simulink model "TosAeb"  (R2021a)
settings: Solver=FixedStepDiscrete  StartTime=0.0  StopTime=inf  FixedStep=0.01  RelTol=1e-3
undefined symbols (not defined in the file or in its folder): ACCModSts, AccSts1, AEB_ObjectMovingOrientation, AEB_ObjectMovingState, CN_BTCActive, CN_CurrentGearR, CN_CurrentGearR_DHT, CN_PTCActive, CN_VDCActive, CN_VSC_EspOff, CN_VSC_FCW_AEB_Error, CN_VSC_FCW_AEB_NoDispay, CN_VSC_FCW_AEB_PerfmDgdtn, CN_VSC_FCW_AEB_TempUnAvlbl, CN_VSC_VehStandstill, CNaccelAvoidMax, CNaccelAvoidMin, CNbrakePedalPress, CNcomfortStrLeftAccelMax, CNcomfortStrLeftAccelMin, CNcomfortStrRightAccelMax, CNcomfortStrRightAccelMin, CNDrvDoorOpen, CNDrvModeEco, CNDrvModeNorm, CNDrvModeOther, CNDrvModeSport, CNDrvSBRreminder, CNNull, CNobjInvalidID, CNPassDoorOpen, CNPassSBRreminder, CNPEBSAccelNegInf, CNPEBSAccelNull, CNPEBSTimeInf, CNPEBSTimeNull, CNSTOPMAX, CNSTOPNULL, CNsubjWidth, CNSwtOFF, CNSwtON, CNTTCMAX, CNTTCNULL, CNVehConfigAEBCycNone, CNVehConfigAEBNone, CNVehConfigAEBPedNone, CNVehConfigFCWNone, CNVehConfigJANone, CollisionSeverity, FALSE, FCWSnvty, JACycObjCalcInfoDefaultParams, JAPedObjCalcInfoDefaultParams, JAVehObjCalcInfoDefaultParams, latCycObjCalcInfoDefaultParams, latPedObjCalcInfoDefaultParams, longObjCalcInfoDefaultParams, ModSts, movingStatus, ObjectType ... (+1112)
libraries: simulink x3031, AEBSFunctionLib x50, Ta_Library x33, AEBSStateMachineLib x23, Mylibrary x12, simulink_extras x7, ObjSelect_lib x3, dspstat3 x2
(part 46 of 102)

== system TosAeb/FcwAebJa_Step_sys/ALG_AEBSystem/ALG_AEBController/AEB_FuncImpManagementApp/FuncImpArbCalc/BrkReadyFuncImpArbCalc/BrkReadyFuncImpArbCalc/FCTB_PrefillFuncImpArbCalc ==
  FCTB_PrefillModStsArb : Inport
  FCTB_PrefillFuncImpAborCond : Inport  Port=2
  Compare To Constant : simulink/Logic and Bit Operations/Compare To Constant  relop=~=  const=ModSts.ActiveState  OutDataTypeStr=boolean
  Compare To Constant1 : simulink/Logic and Bit Operations/Compare To Constant  relop===  const=FALSE  OutDataTypeStr=boolean
  Constant : Constant  Value=ModSts.StandbyState
  Switch : Switch  Criteria=u2 ~= 0
  Switch1 : Switch  Criteria=u2 ~= 0
  FCTB_PrefillFuncImpArb : Outport
  wires:
  FCTB_PrefillModStsArb/1 -> Switch1/1, Switch/1, Compare To Constant/1
  Compare To Constant/1 -> Switch/2
  FCTB_PrefillFuncImpAborCond/1 -> Compare To Constant1/1
  Compare To Constant1/1 -> Switch1/2
  Constant/1 -> Switch1/3
  Switch1/1 -> Switch/3
  Switch/1 -> FCTB_PrefillFuncImpArb/1

== system TosAeb/FcwAebJa_Step_sys/ALG_AEBSystem/ALG_AEBController/AEB_FuncImpManagementApp/FuncImpArbCalc/BrkReadyFuncImpArbCalc/BrkReadyFuncImpArbCalc/JA_PTW_PrefillFuncImpArbCalc ==
  JA_PTW_PrefillModStsArb : Inport
  JA_PTW_PrefillFuncImpAborCond : Inport  Port=2
  Compare To Constant : simulink/Logic and Bit Operations/Compare To Constant  relop=~=  const=ModSts.ActiveState  OutDataTypeStr=boolean
  Compare To Constant1 : simulink/Logic and Bit Operations/Compare To Constant  relop===  const=FALSE  OutDataTypeStr=boolean
  Constant : Constant  Value=ModSts.StandbyState
  Switch : Switch  Criteria=u2 ~= 0
  Switch1 : Switch  Criteria=u2 ~= 0
  JA_PTW_PrefillFuncImpArb : Outport
  wires:
  Switch/1 -> JA_PTW_PrefillFuncImpArb/1
  Switch1/1 -> Switch/3
  Constant/1 -> Switch1/3
  Compare To Constant1/1 -> Switch1/2
  JA_PTW_PrefillFuncImpAborCond/1 -> Compare To Constant1/1
  Compare To Constant/1 -> Switch/2
  JA_PTW_PrefillModStsArb/1 -> Compare To Constant/1, Switch/1, Switch1/1

== system TosAeb/FcwAebJa_Step_sys/ALG_AEBSystem/ALG_AEBController/AEB_FuncImpManagementApp/FuncImpArbCalc/BrkReadyFuncImpArbCalc/BrkReadyFuncImpArbCalc/JA_cycPrefillFuncImpArbCalc ==
  JA_cycPrefillModStsArb : Inport
  JA_cycPrefillFuncImpAborCond : Inport  Port=2
  Compare To Constant : simulink/Logic and Bit Operations/Compare To Constant  relop=~=  const=ModSts.ActiveState  OutDataTypeStr=boolean
  Compare To Constant1 : simulink/Logic and Bit Operations/Compare To Constant  relop===  const=FALSE  OutDataTypeStr=boolean
  Constant : Constant  Value=ModSts.StandbyState
  Switch : Switch  Criteria=u2 ~= 0
  Switch1 : Switch  Criteria=u2 ~= 0
  JA_cycPrefillFuncImpArb : Outport
  wires:
  JA_cycPrefillModStsArb/1 -> Switch1/1, Switch/1, Compare To Constant/1
  Compare To Constant/1 -> Switch/2
  JA_cycPrefillFuncImpAborCond/1 -> Compare To Constant1/1
  Compare To Constant1/1 -> Switch1/2
  Constant/1 -> Switch1/3
  Switch1/1 -> Switch/3
  Switch/1 -> JA_cycPrefillFuncImpArb/1

== system TosAeb/FcwAebJa_Step_sys/ALG_AEBSystem/ALG_AEBController/AEB_FuncImpManagementApp/FuncImpArbCalc/BrkReadyFuncImpArbCalc/BrkReadyFuncImpArbCalc/JA_pedPrefillFuncImpArbCalc ==
  JA_pedPrefillModStsArb : Inport
  JA_pedPrefillFuncImpAborCond : Inport  Port=2
  Compare To Constant : simulink/Logic and Bit Operations/Compare To Constant  relop=~=  const=ModSts.ActiveState  OutDataTypeStr=boolean
  Compare To Constant1 : simulink/Logic and Bit Operations/Compare To Constant  relop===  const=FALSE  OutDataTypeStr=boolean
  Constant : Constant  Value=ModSts.StandbyState
  Switch : Switch  Criteria=u2 ~= 0
  Switch1 : Switch  Criteria=u2 ~= 0
  JA_pedPrefillFuncImpArb : Outport
  wires:
  Switch/1 -> JA_pedPrefillFuncImpArb/1
  Switch1/1 -> Switch/3
  Constant/1 -> Switch1/3
  Compare To Constant1/1 -> Switch1/2
  JA_pedPrefillFuncImpAborCond/1 -> Compare To Constant1/1
  Compare To Constant/1 -> Switch/2
  JA_pedPrefillModStsArb/1 -> Compare To Constant/1, Switch/1, Switch1/1

== system TosAeb/FcwAebJa_Step_sys/ALG_AEBSystem/ALG_AEBController/AEB_FuncImpManagementApp/FuncImpArbCalc/BrkReadyFuncImpArbCalc/BrkReadyFuncImpArbCalc/JA_vehPrefillFuncImpArbCalc ==
  JA_vehPrefillModStsArb : Inport
  JA_vehPrefillFuncImpAborCond : Inport  Port=2
  Compare To Constant : simulink/Logic and Bit Operations/Compare To Constant  relop=~=  const=ModSts.ActiveState  OutDataTypeStr=boolean
  Compare To Constant1 : simulink/Logic and Bit Operations/Compare To Constant  relop===  const=FALSE  OutDataTypeStr=boolean
  Constant : Constant  Value=ModSts.StandbyState
  Switch : Switch  Criteria=u2 ~= 0
  Switch1 : Switch  Criteria=u2 ~= 0
  JA_vehPrefillFuncImpArb : Outport
  wires:
  JA_vehPrefillModStsArb/1 -> Switch1/1, Switch/1, Compare To Constant/1
  Compare To Constant/1 -> Switch/2
  JA_vehPrefillFuncImpAborCond/1 -> Compare To Constant1/1
  Compare To Constant1/1 -> Switch1/2
  Constant/1 -> Switch1/3
  Switch1/1 -> Switch/3
  Switch/1 -> JA_vehPrefillFuncImpArb/1

== system TosAeb/FcwAebJa_Step_sys/ALG_AEBSystem/ALG_AEBController/AEB_FuncImpManagementApp/FuncImpArbCalc/BrkReadyFuncImpArbCalc/BrkReadyFuncImpArbCalc/PrefillFuncImpArbCalc ==
  prefillModStsArb : Inport
  prefillFuncImpAborCond : Inport  Port=2
  Compare To Constant : simulink/Logic and Bit Operations/Compare To Constant  relop=~=  const=ModSts.ActiveState  OutDataTypeStr=boolean
  Compare To Constant1 : simulink/Logic and Bit Operations/Compare To Constant  relop===  const=FALSE  OutDataTypeStr=boolean
  Constant : Constant  Value=ModSts.StandbyState
  Switch : Switch  Criteria=u2 ~= 0
  Switch1 : Switch  Criteria=u2 ~= 0
  prefillFuncImpArb : Outport
  wires:
  Switch/1 -> prefillFuncImpArb/1
  Switch1/1 -> Switch/3
  Constant/1 -> Switch1/3
  Compare To Constant1/1 -> Switch1/2
  prefillFuncImpAborCond/1 -> Compare To Constant1/1
  Compare To Constant/1 -> Switch/2
  prefillModStsArb/1 -> Compare To Constant/1, Switch/1, Switch1/1

== system TosAeb/FcwAebJa_Step_sys/ALG_AEBSystem/ALG_AEBController/AEB_FuncImpManagementApp/FuncImpArbCalc/BrkReadyFuncImpArbCalc/BrkReadyFuncImpArbCalc/cycPrefillFuncImpArbCalc ==
  cycPrefillModStsArb : Inport
  cycPrefillFuncImpAborCond : Inport  Port=2
  Compare To Constant : simulink/Logic and Bit Operations/Compare To Constant  relop=~=  const=ModSts.ActiveState  OutDataTypeStr=boolean
  Compare To Constant1 : simulink/Logic and Bit Operations/Compare To Constant  relop===  const=FALSE  OutDataTypeStr=boolean
  Constant : Constant  Value=ModSts.StandbyState
  Switch : Switch  Criteria=u2 ~= 0
  Switch1 : Switch  Criteria=u2 ~= 0
  cycPrefillFuncImpArb : Outport
  wires:
  Switch/1 -> cycPrefillFuncImpArb/1
  Switch1/1 -> Switch/3
  Constant/1 -> Switch1/3
  Compare To Constant1/1 -> Switch1/2
  cycPrefillFuncImpAborCond/1 -> Compare To Constant1/1
  Compare To Constant/1 -> Switch/2
  cycPrefillModStsArb/1 -> Compare To Constant/1, Switch/1, Switch1/1

== system TosAeb/FcwAebJa_Step_sys/ALG_AEBSystem/ALG_AEBController/AEB_FuncImpManagementApp/FuncImpArbCalc/BrkReadyFuncImpArbCalc/BrkReadyFuncImpArbCalc/pedABAFuncImpArbCalc ==
  pedABAModStsArb : Inport
  pedABAFuncImpAborCond : Inport  Port=2
  Compare To Constant : simulink/Logic and Bit Operations/Compare To Constant  relop=~=  const=ModSts.ActiveState  OutDataTypeStr=boolean
  Compare To Constant1 : simulink/Logic and Bit Operations/Compare To Constant  relop===  const=FALSE  OutDataTypeStr=boolean
  Constant : Constant  Value=ModSts.StandbyState
  Switch : Switch  Criteria=u2 ~= 0
  Switch1 : Switch  Criteria=u2 ~= 0
  pedABAFuncImpArb : Outport
  wires:
  pedABAModStsArb/1 -> Switch1/1, Switch/1, Compare To Constant/1
  Compare To Constant/1 -> Switch/2
  pedABAFuncImpAborCond/1 -> Compare To Constant1/1
  Compare To Constant1/1 -> Switch1/2
  Constant/1 -> Switch1/3
  Switch1/1 -> Switch/3
  Switch/1 -> pedABAFuncImpArb/1

== system TosAeb/FcwAebJa_Step_sys/ALG_AEBSystem/ALG_AEBController/AEB_FuncImpManagementApp/FuncImpArbCalc/BrkReadyFuncImpArbCalc/BrkReadyFuncImpArbCalc/pedPrefillFuncImpArbCalc ==
  pedPrefillModStsArb : Inport
  pedPrefillFuncImpAborCond : Inport  Port=2
  Compare To Constant : simulink/Logic and Bit Operations/Compare To Constant  relop=~=  const=ModSts.ActiveState  OutDataTypeStr=boolean
  Compare To Constant1 : simulink/Logic and Bit Operations/Compare To Constant  relop===  const=FALSE  OutDataTypeStr=boolean
  Constant : Constant  Value=ModSts.StandbyState
  Switch : Switch  Criteria=u2 ~= 0
  Switch1 : Switch  Criteria=u2 ~= 0
  pedPrefillFuncImpArb : Outport
  wires:
  Switch/1 -> pedPrefillFuncImpArb/1
  Switch1/1 -> Switch/3
  Constant/1 -> Switch1/3
  Compare To Constant1/1 -> Switch1/2
  pedPrefillFuncImpAborCond/1 -> Compare To Constant1/1
  Compare To Constant/1 -> Switch/2
  pedPrefillModStsArb/1 -> Compare To Constant/1, Switch/1, Switch1/1

== system TosAeb/FcwAebJa_Step_sys/ALG_AEBSystem/ALG_AEBController/AEB_FuncImpManagementApp/FuncImpArbCalc/BrkReadyFuncImpArbCalc1/BrkReadyFuncImpArbCalc/ABAFuncImpArbCalc ==
  ABAModStsArb : Inport
  ABAFuncImpAborCond : Inport  Port=2
  Compare To Constant : simulink/Logic and Bit Operations/Compare To Constant  relop=~=  const=ModSts.ActiveState  OutDataTypeStr=boolean
  Compare To Constant1 : simulink/Logic and Bit Operations/Compare To Constant  relop===  const=FALSE  OutDataTypeStr=boolean
  Constant : Constant  Value=ModSts.StandbyState
  Switch : Switch  Criteria=u2 ~= 0
  Switch1 : Switch  Criteria=u2 ~= 0
  ABAFuncImpArb : Outport
  wires:
  Switch/1 -> ABAFuncImpArb/1
  Switch1/1 -> Switch/3
  Constant/1 -> Switch1/3
  Compare To Constant1/1 -> Switch1/2
  ABAFuncImpAborCond/1 -> Compare To Constant1/1
  Compare To Constant/1 -> Switch/2
  ABAModStsArb/1 -> Compare To Constant/1, Switch/1, Switch1/1

== system TosAeb/FcwAebJa_Step_sys/ALG_AEBSystem/ALG_AEBController/AEB_FuncImpManagementApp/FuncImpArbCalc/BrkReadyFuncImpArbCalc1/BrkReadyFuncImpArbCalc/JA_vehPrefillFuncImpArbCalc ==
  JA_vehPrefillModStsArb : Inport
  JA_vehPrefillFuncImpAborCond : Inport  Port=2
  Compare To Constant : simulink/Logic and Bit Operations/Compare To Constant  relop=~=  const=ModSts.ActiveState  OutDataTypeStr=boolean
  Compare To Constant1 : simulink/Logic and Bit Operations/Compare To Constant  relop===  const=FALSE  OutDataTypeStr=boolean
  Constant : Constant  Value=ModSts.StandbyState
  Switch : Switch  Criteria=u2 ~= 0
  Switch1 : Switch  Criteria=u2 ~= 0
  JA_vehPrefillFuncImpArb : Outport
  wires:
  Switch/1 -> JA_vehPrefillFuncImpArb/1
  Switch1/1 -> Switch/3
  Constant/1 -> Switch1/3
  Compare To Constant1/1 -> Switch1/2
  JA_vehPrefillFuncImpAborCond/1 -> Compare To Constant1/1
  Compare To Constant/1 -> Switch/2
  JA_vehPrefillModStsArb/1 -> Compare To Constant/1, Switch/1, Switch1/1

== system TosAeb/FcwAebJa_Step_sys/ALG_AEBSystem/ALG_AEBController/AEB_FuncImpManagementApp/FuncImpArbCalc/BrkReadyFuncImpArbCalc1/BrkReadyFuncImpArbCalc/PrefillFuncImpArbCalc ==
  prefillModStsArb : Inport
  prefillFuncImpAborCon d : Inport  Port=2
  Compare To Constant : simulink/Logic and Bit Operations/Compare To Constant  relop=~=  const=ModSts.ActiveState  OutDataTypeStr=boolean
  Compare To Constant1 : simulink/Logic and Bit Operations/Compare To Constant  relop===  const=FALSE  OutDataTypeStr=boolean
  Constant : Constant  Value=ModSts.StandbyState
  Switch : Switch  Criteria=u2 ~= 0
  Switch1 : Switch  Criteria=u2 ~= 0
  prefillFuncImpArb : Outport
  wires:
  prefillModStsArb/1 -> Switch1/1, Switch/1, Compare To Constant/1
  Compare To Constant/1 -> Switch/2
  prefillFuncImpAborCon d/1 -> Compare To Constant1/1
  Compare To Constant1/1 -> Switch1/2
  Constant/1 -> Switch1/3
  Switch1/1 -> Switch/3
  Switch/1 -> prefillFuncImpArb/1

== system TosAeb/FcwAebJa_Step_sys/ALG_AEBSystem/ALG_AEBController/AEB_FuncImpManagementApp/FuncImpArbCalc/BrkReadyFuncImpArbCalc1/BrkReadyFuncImpArbCalc/cycPrefillFuncImpArbCalc ==
  cycPrefillModStsArb : Inport
  cycPrefillFuncImpAborCond : Inport  Port=2
  Compare To Constant : simulink/Logic and Bit Operations/Compare To Constant  relop=~=  const=ModSts.ActiveState  OutDataTypeStr=boolean
  Compare To Constant1 : simulink/Logic and Bit Operations/Compare To Constant  relop===  const=FALSE  OutDataTypeStr=boolean
  Constant : Constant  Value=ModSts.StandbyState
  Switch : Switch  Criteria=u2 ~= 0
  Switch1 : Switch  Criteria=u2 ~= 0
  cycPrefillFuncImpArb : Outport
  wires:
  cycPrefillModStsArb/1 -> Switch1/1, Switch/1, Compare To Constant/1
  Compare To Constant/1 -> Switch/2
  cycPrefillFuncImpAborCond/1 -> Compare To Constant1/1
  Compare To Constant1/1 -> Switch1/2
  Constant/1 -> Switch1/3
  Switch1/1 -> Switch/3
  Switch/1 -> cycPrefillFuncImpArb/1

== system TosAeb/FcwAebJa_Step_sys/ALG_AEBSystem/ALG_AEBController/AEB_FuncImpManagementApp/FuncImpArbCalc/BrkReadyFuncImpArbCalc1/BrkReadyFuncImpArbCalc/pedABAFuncImpArbCalc ==
  pedABAModStsArb : Inport
  pedABAFuncImpAborCond : Inport  Port=2
  Compare To Constant : simulink/Logic and Bit Operations/Compare To Constant  relop=~=  const=ModSts.ActiveState  OutDataTypeStr=boolean
  Compare To Constant1 : simulink/Logic and Bit Operations/Compare To Constant  relop===  const=FALSE  OutDataTypeStr=boolean
  Constant : Constant  Value=ModSts.StandbyState
  Switch : Switch  Criteria=u2 ~= 0
  Switch1 : Switch  Criteria=u2 ~= 0
  pedABAFuncImpArb : Outport
  wires:
  Switch/1 -> pedABAFuncImpArb/1
  Switch1/1 -> Switch/3
  Constant/1 -> Switch1/3
  Compare To Constant1/1 -> Switch1/2
  pedABAFuncImpAborCond/1 -> Compare To Constant1/1
  Compare To Constant/1 -> Switch/2
  pedABAModStsArb/1 -> Compare To Constant/1, Switch/1, Switch1/1

== system TosAeb/FcwAebJa_Step_sys/ALG_AEBSystem/ALG_AEBController/AEB_FuncImpManagementApp/FuncImpArbCalc/BrkReadyFuncImpArbCalc1/BrkReadyFuncImpArbCalc/pedPrefillFuncImpArbCalc ==
  pedPrefillModStsArb : Inport
  pedPrefillFuncImpAborCond : Inport  Port=2
  Compare To Constant : simulink/Logic and Bit Operations/Compare To Constant  relop=~=  const=ModSts.ActiveState  OutDataTypeStr=boolean
  Compare To Constant1 : simulink/Logic and Bit Operations/Compare To Constant  relop===  const=FALSE  OutDataTypeStr=boolean
  Constant : Constant  Value=ModSts.StandbyState
  Switch : Switch  Criteria=u2 ~= 0
  Switch1 : Switch  Criteria=u2 ~= 0
  pedPrefillFuncImpArb : Outport
  wires:
  pedPrefillModStsArb/1 -> Switch1/1, Switch/1, Compare To Constant/1
  Compare To Constant/1 -> Switch/2
  pedPrefillFuncImpAborCond/1 -> Compare To Constant1/1
  Compare To Constant1/1 -> Switch1/2
  Constant/1 -> Switch1/3
  Switch1/1 -> Switch/3
  Switch/1 -> pedPrefillFuncImpArb/1

== system TosAeb/FcwAebJa_Step_sys/ALG_AEBSystem/ALG_AEBController/AEB_FuncImpManagementApp/FuncImpArbCalc/WarningFuncImpArbCalc/WarningFuncImpArbCalc/AcuteFuncImpArbCalc ==
  acuteWarningModStsArb : Inport
  acuteWarningFuncImpAborCond : Inport  Port=2
  Compare To Constant : simulink/Logic and Bit Operations/Compare To Constant  relop=~=  const=ModSts.ActiveState  OutDataTypeStr=boolean
  Compare To Constant1 : simulink/Logic and Bit Operations/Compare To Constant  relop===  const=FALSE  OutDataTypeStr=boolean
  Constant : Constant  Value=ModSts.StandbyState
  Switch : Switch  Criteria=u2 ~= 0
  Switch1 : Switch  Criteria=u2 ~= 0
  acuteWarningFuncImpArb : Outport
  wires:
  Switch/1 -> acuteWarningFuncImpArb/1
  Switch1/1 -> Switch/3
  Constant/1 -> Switch1/3
  Compare To Constant1/1 -> Switch1/2
  acuteWarningFuncImpAborCond/1 -> Compare To Constant1/1
  Compare To Constant/1 -> Switch/2
  acuteWarningModStsArb/1 -> Compare To Constant/1, Switch/1, Switch1/1

== system TosAeb/FcwAebJa_Step_sys/ALG_AEBSystem/ALG_AEBController/AEB_FuncImpManagementApp/FuncImpArbCalc/WarningFuncImpArbCalc/WarningFuncImpArbCalc/LatentWarningFuncImpArbCalc ==
  latentWarningModStsArb : Inport
  latentWarningFuncImpAborCond : Inport  Port=2
  Compare To Constant : simulink/Logic and Bit Operations/Compare To Constant  relop=~=  const=ModSts.ActiveState  OutDataTypeStr=boolean
  Compare To Constant1 : simulink/Logic and Bit Operations/Compare To Constant  relop===  const=FALSE  OutDataTypeStr=boolean
  Constant : Constant  Value=ModSts.StandbyState
  Switch : Switch  Criteria=u2 ~= 0
  Switch1 : Switch  Criteria=u2 ~= 0
  latentWarningFuncImpArb : Outport
  wires:
  Switch/1 -> latentWarningFuncImpArb/1
  Switch1/1 -> Switch/3
  Constant/1 -> Switch1/3
  Compare To Constant1/1 -> Switch1/2
  latentWarningFuncImpAborCond/1 -> Compare To Constant1/1
  Compare To Constant/1 -> Switch/2
  latentWarningModStsArb/1 -> Compare To Constant/1, Switch/1, Switch1/1

== system TosAeb/FcwAebJa_Step_sys/ALG_AEBSystem/ALG_AEBController/AEB_FuncImpManagementApp/FuncImpArbCalc/WarningFuncImpArbCalc/WarningFuncImpArbCalc/PreWarningFuncImpArbCalc ==
  preWarningModStsArb : Inport
  preWarningFuncImpAborCond : Inport  Port=2
  Compare To Constant : simulink/Logic and Bit Operations/Compare To Constant  relop=~=  const=ModSts.ActiveState  OutDataTypeStr=boolean
  Compare To Constant1 : simulink/Logic and Bit Operations/Compare To Constant  relop===  const=FALSE  OutDataTypeStr=boolean
  Constant : Constant  Value=ModSts.StandbyState
  Switch : Switch  Criteria=u2 ~= 0
  Switch1 : Switch  Criteria=u2 ~= 0
  preWarningFuncImpArb : Outport
  wires:
  preWarningModStsArb/1 -> Switch1/1, Switch/1, Compare To Constant/1
  Compare To Constant/1 -> Switch/2
  preWarningFuncImpAborCond/1 -> Compare To Constant1/1
  Compare To Constant1/1 -> Switch1/2
  Constant/1 -> Switch1/3
  Switch1/1 -> Switch/3
  Switch/1 -> preWarningFuncImpArb/1

== system TosAeb/FcwAebJa_Step_sys/ALG_AEBSystem/ALG_AEBController/AEB_FuncImpManagementApp/FuncImpArbCalc/WarningFuncImpArbCalc/WarningFuncImpArbCalc/pedPreWarningFuncImpArbCalc ==
  pedPreWarningModStsArb : Inport
  pedPreWarningFuncImpAborCond : Inport  Port=2
  Compare To Constant : simulink/Logic and Bit Operations/Compare To Constant  relop=~=  const=ModSts.ActiveState  OutDataTypeStr=boolean
  Compare To Constant1 : simulink/Logic and Bit Operations/Compare To Constant  relop===  const=FALSE  OutDataTypeStr=boolean
  Constant : Constant  Value=ModSts.StandbyState
  Switch : Switch  Criteria=u2 ~= 0
  Switch1 : Switch  Criteria=u2 ~= 0
  pedPreWarningFuncImpArb : Outport
  wires:
  pedPreWarningModStsArb/1 -> Switch1/1, Switch/1, Compare To Constant/1
  Compare To Constant/1 -> Switch/2
  pedPreWarningFuncImpAborCond/1 -> Compare To Constant1/1
  Compare To Constant1/1 -> Switch1/2
  Constant/1 -> Switch1/3
  Switch1/1 -> Switch/3
  Switch/1 -> pedPreWarningFuncImpArb/1

== system TosAeb/FcwAebJa_Step_sys/ALG_AEBSystem/ALG_AEBController/AEB_FuncImpManagementApp/FuncManeuArbCalc/BrkReadyManeuArbCalc/BrkReadyTriggerJudge/ABALvlCalc ==
  ABATrigger : Inport
  ABAFuncImpArb : Inport  Port=2
  pedABAFuncImpArb : Inport  Port=3
  FCTB_ABAFuncImpArb : Inport  Port=4
  longCollisionSeverity : Inport  Port=5
  pedCollisionSeverity : Inport  Port=6
  FCTB_ABA_Level : Inport  Port=7
  Compare To Constant1 : simulink/Logic and Bit Operations/Compare To Constant  relop===  const=ModSts.ActiveState  OutDataTypeStr=boolean
  Compare To Constant2 : simulink/Logic and Bit Operations/Compare To Constant  relop===  const=ModSts.ActiveState  OutDataTypeStr=boolean
  Compare To Constant5 : simulink/Logic and Bit Operations/Compare To Constant  relop===  const=ModSts.ActiveState  OutDataTypeStr=boolean
  If : If  NumInputs=2  IfExpression=u1  ElseIfExpressions=u2
  If Action Subsystem : Subsystem  TreatAsAtomicUnit=on
  If Action Subsystem1 : Subsystem  TreatAsAtomicUnit=on
  If Action Subsystem2 : Subsystem  TreatAsAtomicUnit=on
  Logical Operator : Logic  Operator=OR  AllPortsSameDT=off  OutDataTypeStr=boolean
  Merge : Merge  Inputs=3
  ABALvl : Outport
  wires:
  [pedABAFuncImpArb] -> If Action Subsystem/4(pedABAFuncImpArb)
  [Default] -> If Action Subsystem2/Action
  If/3 -> [Default]
  [FCTB_ABA] -> If Action Subsystem1/Action
  If/2 -> [FCTB_ABA]
  FCTB_ABA_Level/1 -> If Action Subsystem1/2(FCTB_ABA_Level)
  Compare To Constant2/1 -> If/2
  ABATrigger/1 -> If Action Subsystem/1(ABATrigger), If Action Subsystem1/1(ABATrigger)
  pedCollisionSeverity/1 -> If Action Subsystem/3(pedCollisionSeverity)
  longCollisionSeverity/1 -> If Action Subsystem/2(longCollisionSeverity)
  Merge/1 -> ABALvl/1
  If Action Subsystem2/1(ABALvl) -> Merge/3
  If Action Subsystem1/1(ABALvl) -> Merge/2
  If Action Subsystem/1(ABALvl) -> Merge/1
  If/1 -> If Action Subsystem/Action
  FCTB_ABAFuncImpArb/1 -> Compare To Constant2/1
  Logical Operator/1 -> If/1
  Compare To Constant1/1 -> Logical Operator/2
  Compare To Constant5/1 -> Logical Operator/1
  pedABAFuncImpArb/1 -> Compare To Constant1/1, [pedABAFuncImpArb]
  ABAFuncImpArb/1 -> Compare To Constant5/1

== system TosAeb/FcwAebJa_Step_sys/ALG_AEBSystem/ALG_AEBController/AEB_FuncImpManagementApp/FuncManeuArbCalc/BrkReadyManeuArbCalc/BrkReadyTriggerJudge/ABATriggerCalc ==
  ABAAvailable : Inport
  ABAFuncImpArb : Inport  Port=2
  pedABAFuncImpArb : Inport  Port=3
  FCTB_ABAFuncImpArb : Inport  Port=4
  Compare To Constant4 : simulink/Logic and Bit Operations/Compare To Constant  relop===  const=ModSts.ActiveState  OutDataTypeStr=boolean
  Compare To Constant5 : simulink/Logic and Bit Operations/Compare To Constant  relop===  const=ModSts.ActiveState  OutDataTypeStr=boolean
  Compare To Constant9 : simulink/Logic and Bit Operations/Compare To Constant  relop===  const=ModSts.ActiveState  OutDataTypeStr=boolean
  Logical Operator1 : Logic  Operator=OR  Inputs=3  AllPortsSameDT=off  OutDataTypeStr=boolean
  Logical Operator2 : Logic  AllPortsSameDT=off  OutDataTypeStr=boolean
  ABATrigger : Outport
  wires:
  FCTB_ABAFuncImpArb/1 -> Compare To Constant9/1
  pedABAFuncImpArb/1 -> Compare To Constant4/1
  ABAFuncImpArb/1 -> Compare To Constant5/1
  Compare To Constant9/1 -> Logical Operator1/3
  Logical Operator2/1 -> ABATrigger/1
  ABAAvailable/1 -> Logical Operator2/1
  Logical Operator1/1 -> Logical Operator2/2
  Compare To Constant5/1 -> Logical Operator1/1
  Compare To Constant4/1 -> Logical Operator1/2

== system TosAeb/FcwAebJa_Step_sys/ALG_AEBSystem/ALG_AEBController/AEB_FuncImpManagementApp/FuncManeuArbCalc/BrkReadyManeuArbCalc/BrkReadyTriggerJudge/ABPTriggerCalc ==
  ABPAvailable : Inport
  prefillFuncImpArb : Inport  Port=2
  pedPrefillFuncImpArb : Inport  Port=3
  cycPrefillFuncImpArb : Inport  Port=4
  JA_vehPrefillFuncImpArb : Inport  Port=5
  JA_pedPrefillFuncImpArb : Inport  Port=6
  JA_cycPedfillFuncImpArb : Inport  Port=7
  Compare To Constant{,1..3,6..7} (6 alike) : simulink/Logic and Bit Operations/Compare To Constant  relop===  const=ModSts.ActiveState  OutDataTypeStr=boolean
  Logical Operator : Logic  Operator=OR  Inputs=6  AllPortsSameDT=off  OutDataTypeStr=boolean
  Logical Operator3 : Logic  AllPortsSameDT=off  OutDataTypeStr=boolean
  ABPTrigger : Outport
  wires:
  JA_cycPedfillFuncImpArb/1 -> Compare To Constant7/1
  JA_pedPrefillFuncImpArb/1 -> Compare To Constant6/1
  JA_vehPrefillFuncImpArb/1 -> Compare To Constant3/1
  cycPrefillFuncImpArb/1 -> Compare To Constant2/1
  pedPrefillFuncImpArb/1 -> Compare To Constant1/1
  prefillFuncImpArb/1 -> Compare To Constant/1
  ABPAvailable/1 -> Logical Operator3/1
  Compare To Constant/1 -> Logical Operator/1
  Compare To Constant3/1 -> Logical Operator/4
  Compare To Constant1/1 -> Logical Operator/2
  Logical Operator3/1 -> ABPTrigger/1
  Logical Operator/1 -> Logical Operator3/2
  Compare To Constant2/1 -> Logical Operator/3
  Compare To Constant6/1 -> Logical Operator/5
  Compare To Constant7/1 -> Logical Operator/6

== system TosAeb/FcwAebJa_Step_sys/ALG_AEBSystem/ALG_AEBController/AEB_FuncImpManagementApp/FuncManeuArbCalc/brkFuncManeuArbCalc/axTgtReqValueCalc1/AebfullTriggerInfoCalc ==
  AEBfullFuncImpArb : Inport  OutDataTypeStr=Enum: ModSts
  VSC_VehStandstill : Inport  Port=2
  subjVel : Inport  Port=3  OutDataTypeStr=single
  dxFrontBumper : Inport  Port=4
  vx : Inport  Port=5
  vxAbsObj : Inport  Port=6
  axAbsObj : Inport  Port=7
  ax : Inport  Port=8
  ObjectMovingState : Inport  Port=9
  AEBControllerInfo : Subsystem
  AEBTriggerRelCalc : Subsystem
  AebActive : Subsystem
  BrakeInfo : Subsystem
  Bus Creator1 : BusCreator  Inputs=7  OutDataTypeStr=Bus: BusFIM_BrkTriggerInfo  NonVirtualBus=on
      settings: InheritFromInputs=off
  Bus Creator2 : BusCreator  Inputs=3  OutDataTypeStr=Bus: BusFIM_BrakeInfo4Control  NonVirtualBus=on
      settings: InheritFromInputs=off
  Bus Selector1 : BusSelector  OutputSignals=vxPredicted
  LowPassFilter : Subsystem  mask{FiltCoef=P_AEB_FIM_FiltCeof4vxAbsObj}
  Subsystem : Subsystem
  axAbsObjFiltCalc : Subsystem
  AebTriggerInfo : Outport  OutDataTypeStr=Bus: BusFIM_BrkTriggerInfo
  ObjectPredicted : Outport  Port=2  OutDataTypeStr=Bus: BusFIM_ObjPredicted
  AEB_FIM_BrakeInfo : Outport  Port=3  OutDataTypeStr=Bus: BusFIM_BrakeInfo4Control
  axAbsObjFilt : Outport  Port=4
  wires:
  BrakeInfo/1(accelMin) -> AEBControllerInfo/2(accelMinIn)
  BrakeInfo/2(JerkFuncDecMax) -> AEBControllerInfo/3(JerkFuncDecMaxIn)
  [subjVel] -> BrakeInfo/1(subjVel)
  ObjectMovingState/1 -> axAbsObjFiltCalc/1(ObjectMovingState), BrakeInfo/2(ObjectMovingState)
  Bus Creator2/1 -> AEB_FIM_BrakeInfo/1  "AEB_FIM_BrakeInfo4Control"
  AEBControllerInfo/1(accelMin) -> Bus Creator2/1  "accelMin"
  AEBControllerInfo/3(JerkFuncDecMax) -> Bus Creator2/3  "GradientFuncDecMax"
  AEBControllerInfo/2(accelMax) -> Bus Creator2/2  "accelMax"
  Bus Selector1/1 -> AEBTriggerRelCalc/2(vxPredicted)  "<vxPredicted>"
  Bus Creator1/1 -> AebTriggerInfo/1  "AEB_AebFullBrkTriggerInfo"
  AEBTriggerRelCalc/7(vxPredictedAtStart) -> AEBControllerInfo/1(vxPredictedAtStart), Bus Creator1/7  "vxPredictedAtStart"
  AEBTriggerRelCalc/5(subjVelAtStart) -> Bus Creator1/5  "subjVelAtStart"
  AEBTriggerRelCalc/6(dxSecureAtStart) -> Bus Creator1/6  "dxSecureAtStart"
  AEBTriggerRelCalc/4(FuncActvationTimeAtStart) -> Bus Creator1/4  "FuncActvationTimeAtStart"
  AEBTriggerRelCalc/1(FuncActvationTime) -> Bus Creator1/1  "FuncActvationTime"
  [subjVel] -> AEBTriggerRelCalc/4(subjVel)
  AebActive/1(AebActive) -> Subsystem/1(AebActive), AEBTriggerRelCalc/1(AebActive)
  AEBfullFuncImpArb/1 -> AebActive/1(AEBfullFuncImpArb)
  VSC_VehStandstill/1 -> AEBTriggerRelCalc/3(VSC_VehStandstill)  "VSC_VehStandstill"
  Subsystem/1(AEB_FIM_ObjectPredicted) -> Bus Selector1/1, ObjectPredicted/1
  subjVel/1 -> [subjVel], Subsystem/2(subjVel)
  dxFrontBumper/1 -> Subsystem/3(dxFrontBumper)
  vx/1 -> Subsystem/4(vx)  "vx"
  vxAbsObj/1 -> LowPassFilter/1(x)
  ax/1 -> Subsystem/6(ax)  "ax"
  AEBTriggerRelCalc/2(AebFullFuncStatus) -> Bus Creator1/2  "AebFullFuncStatus"
  AEBTriggerRelCalc/3(AebActiveFirstCycle) -> Bus Creator1/3  "AebActiveFirstCycle"
  axAbsObjFiltCalc/1(axAbsObjFilt) -> axAbsObjFilt/1  "axAbsObjFilt"
  axAbsObj/1 -> axAbsObjFiltCalc/2(axAbsObj)
  LowPassFilter/1(y) -> Subsystem/5(vxAbsObj)  "vxAbsObjPredicted"

== system TosAeb/FcwAebJa_Step_sys/ALG_AEBSystem/ALG_AEBController/AEB_FuncImpManagementApp/FuncManeuArbCalc/brkFuncManeuArbCalc/axTgtReqValueCalc1/Fctb_IsNeedBrakeCalc ==
  FctbReq : Inport  OutDataTypeStr=Enum: ModSts
  VehicleStandstill : Inport  Port=2  OutDataTypeStr=uint8
  subjVel : Inport  Port=3  OutDataTypeStr=single
  FCTB_BrkgReqVal : Inport  Port=4
  Compare To Constant : simulink/Logic and Bit Operations/Compare To Constant  relop===  const=ModSts.ActiveState  OutDataTypeStr=boolean
  Constant23 : Constant  Value=P_AEB_FIM_FctbDistThes
  IsNeedBrakeFlow : Stateflow chart  TreatAsAtomicUnit=on
  Terminator{,1..3} (4 alike) : Terminator
  IsNeedBrake : Outport
  wires:
  subjVel/1 -> IsNeedBrakeFlow/3(subjVel)
  VehicleStandstill/1 -> IsNeedBrakeFlow/2(standstill)  "VehicleStandstill"
  FctbReq/1 -> Compare To Constant/1
  IsNeedBrakeFlow/1(IsNeedBrake) -> IsNeedBrake/1
  Compare To Constant/1 -> IsNeedBrakeFlow/1(FctbReq)
  FCTB_BrkgReqVal/1 -> IsNeedBrakeFlow/4(Fctb_AxReq)
  Constant23/1 -> IsNeedBrakeFlow/5(P_AEB_FIM_FctbDistThes)
  IsNeedBrakeFlow/2(FctbState) -> Terminator/1  "AEB_Fctb_State"
  IsNeedBrakeFlow/4(Distance1) -> Terminator1/1  "AEB_Fctb_Distance1"
  IsNeedBrakeFlow/5(Distance2) -> Terminator2/1  "AEB_Fctb_Distance2"
  IsNeedBrakeFlow/3(Distance) -> Terminator3/1  "AEB_Fctb_Distance"

== system TosAeb/FcwAebJa_Step_sys/ALG_AEBSystem/ALG_AEBController/AEB_FuncImpManagementApp/FuncManeuArbCalc/brkFuncManeuArbCalc/axTgtReqValueCalc1/axTgtReqValueCalc4AebControl ==
  AebTriggerInfo : Inport  OutDataTypeStr=Bus: BusFIM_BrkTriggerInfo
  ObjectPredicted : Inport  Port=2  OutDataTypeStr=Bus: BusFIM_ObjPredicted
  AEB_FIM_BrakeInfo : Inport  Port=3  OutDataTypeStr=Bus: BusFIM_BrakeInfo4Control
  axAbsObj : Inport  Port=4  OutDataTypeStr=single
  VSC_VehStandstill : Inport  Port=5
  subjVel : Inport  Port=6  OutDataTypeStr=single
  vx : Inport  Port=7  OutDataTypeStr=single
  subjLongAccel : Inport  Port=8
  axTgtReqValueK1 : Inport  Port=9
  Action Port : ActionPort  ActionPortLabel=if(u1)
  Bus Creator : BusCreator  Inputs=9
  Bus Selector : BusSelector  OutputSignals=AebTriggerInfo.AebActiveFirstCycle,subjVel,axAbsObj,ObjectPredicted.dxFrontBumperPredicted,ObjectPredicted.vxAbsObjPredicted,ObjectPredicted.vxPredicted,AebTriggerInfo.dxSecureAtStart,AEB_FIM_BrakeInfo.accelMin
  Bus Selector1 : BusSelector  OutputSignals=axTgtReqValueK1,axAbsObj,ObjectPredicted.dxFrontBumperPredicted,AebTriggerInfo.vxPredictedAtStart,AebTriggerInfo.AebActiveFirstCycle
  Bus Selector2 : BusSelector  OutputSignals=AebTriggerInfo.FuncActvationTime,AebTriggerInfo.AebActiveFirstCycle,AebTriggerInfo.FuncActvationTimeAtStart,AebTriggerInfo.vxPredictedAtStart,AEB_FIM_BrakeInfo.accelMin,AEB_FIM_BrakeInfo.GradientFuncDecMax,axTgtReqValueK1
  Bus Selector3 : BusSelector  OutputSignals=vx,subjLongAccel,axTgtReqValueK1,ObjectPredicted.dxFrontBumperPredicted,AEB_FIM_BrakeInfo.accelMax,AEB_FIM_BrakeInfo.accelMin
  Bus Selector4 : BusSelector  OutputSignals=axTgtReqValueK1,AebTriggerInfo.AebFullFuncStatus
  JerkFuncDecCalc : Subsystem
  JerkFuncIncCalc : Subsystem
  Terminator : Terminator
  Terminator1 : Terminator
  aDesiredCalc : Subsystem
  axTgtReqValueCalc : Subsystem
  axTgtReqValueOpt : Subsystem
  axTgtReqValue : Outport
  wires:
  axTgtReqValueK1/1 -> Bus Creator/9  "axTgtReqValueK1"
  AEB_FIM_BrakeInfo/1 -> Bus Creator/3  "AEB_FIM_BrakeInfo"
  JerkFuncIncCalc/1(ReachedIncStage) -> Terminator1/1  "AEB_FIM_ReachedIncStage"
  aDesiredCalc/1(aDesired) -> axTgtReqValueCalc/8(aDesired), JerkFuncDecCalc/1(aDesired), JerkFuncIncCalc/6(aDesired)  "AEB_FIM_aDesired"
  JerkFuncIncCalc/3(JerkFuncInc) -> axTgtReqValueCalc/7(JerkFuncInc)  "AEB_FIM_GradientFuncInc"
  JerkFuncIncCalc/2(currentIncStage) -> Terminator/1  "AEB_FIM_currentIncStage"
  JerkFuncDecCalc/1(JerkFuncDec) -> axTgtReqValueCalc/9(JerkFuncDec)  "AEB_FIM_GradientFuncDec"
  subjVel/1 -> Bus Creator/5  "subjVel"
  axAbsObj/1 -> Bus Creator/6  "axAbsObj"
  vx/1 -> Bus Creator/7  "vx"
  subjLongAccel/1 -> Bus Creator/8  "subjLongAccel"
  ObjectPredicted/1 -> Bus Creator/2  "ObjectPredicted"
  AebTriggerInfo/1 -> Bus Creator/1  "AebTriggerInfo"
  Bus Creator/1 -> Bus Selector2/1, Bus Selector/1, Bus Selector4/1, Bus Selector3/1, Bus Selector1/1
  Bus Selector/5 -> aDesiredCalc/5(vxAbsObjPredicted)  "<vxAbsObjPredicted>"
  Bus Selector/4 -> aDesiredCalc/4(dxFrontBumperPredicted)  "<dxFrontBumperPredicted>"
  Bus Selector/6 -> aDesiredCalc/6(vxPredicted)  "<vxPredicted>"
  Bus Selector/3 -> aDesiredCalc/3(axAbsObj)  "<axAbsObj>"
  Bus Selector/7 -> aDesiredCalc/7(dxSecureAtStart)  "<dxSecureAtStart>"
  Bus Selector/2 -> aDesiredCalc/2(subjVel)  "<subjVel>"
  Bus Selector/8 -> aDesiredCalc/8(accelMin)  "<accelMin>"
  Bus Selector2/1 -> JerkFuncDecCalc/2(FuncActvationTime)  "<FuncActvationTime>"
  Bus Selector2/3 -> JerkFuncDecCalc/4(FuncActvationTimeAtStart)  "<FuncActvationTimeAtStart>"
  Bus Selector2/4 -> JerkFuncDecCalc/5(vxAtStart)  "<vxPredictedAtStart>"
  Bus Selector2/5 -> JerkFuncDecCalc/6(accelMin)  "<accelMin>"
  Bus Selector2/6 -> JerkFuncDecCalc/7(JerkFuncDecMax)  "<GradientFuncDecMax>"
  Bus Selector2/7 -> JerkFuncDecCalc/8(axTgtReqValueK1)  "<axTgtReqValueK1>"
  Bus Selector1/3 -> JerkFuncIncCalc/3(dxFrontBumperPredicted)  "<dxFrontBumperPredicted>"
  Bus Selector1/4 -> JerkFuncIncCalc/4(vxPredictedAtStart)  "<vxPredictedAtStart>"
  Bus Selector1/1 -> JerkFuncIncCalc/1(axTgtReqValueK1)  "<axTgtReqValueK1>"
  Bus Selector1/2 -> JerkFuncIncCalc/2(axAbsObj)  "<axAbsObj>"
  Bus Selector3/4 -> axTgtReqValueCalc/4(dxFrontBumperPredicted)  "<dxFrontBumperPredicted>"
  Bus Selector3/3 -> axTgtReqValueCalc/3(axTgtReqValueK1)  "<axTgtReqValueK1>"
  Bus Selector3/2 -> axTgtReqValueCalc/2(subjLongAccel)  "<subjLongAccel>"
  Bus Selector3/1 -> axTgtReqValueCalc/1(vx)  "<vx>"
  Bus Selector3/5 -> axTgtReqValueCalc/5(accelMax)  "<accelMax>"
  Bus Selector3/6 -> axTgtReqValueCalc/6(accelMin)  "<accelMin>"
  axTgtReqValueOpt/1(axTgtReqValueOpt) -> axTgtReqValue/1
  VSC_VehStandstill/1 -> Bus Creator/4  "VSC_VehStandstill"
  Bus Selector4/1 -> axTgtReqValueOpt/1(axTgtReqValueK1)  "<axTgtReqValueK1>"
  Bus Selector4/2 -> axTgtReqValueOpt/2(AebFullFuncStatus)  "<AebFullFuncStatus>"
  axTgtReqValueCalc/1(axTgtReqValue) -> axTgtReqValueOpt/3(axTgtReqValue)
  Bus Selector2/2 -> JerkFuncDecCalc/3(AebActiveFirstCycle)  "<AebActiveFirstCycle>"
  Bus Selector1/5 -> JerkFuncIncCalc/5(AebActiveFirstCycle)  "<AebActiveFirstCycle>"
  Bus Selector/1 -> aDesiredCalc/1(AebActiveFirstCycle)  "<AebActiveFirstCycle>"

== system TosAeb/FcwAebJa_Step_sys/ALG_AEBSystem/ALG_AEBController/AEB_FuncImpManagementApp/FuncManeuArbCalc/brkFuncManeuArbCalc/axTgtReqValueCalc1/axTgtReqValueCalc4AebFull ==
  AEB_FctbIsNeedBrake : Inport
  AEBModStsFuncImpArb : Inport  Port=2
  subjVel : Inport  Port=3
  FCTB_BrkgReqVal : Inport  Port=4
  ObjectMovingState : Inport  Port=5
  axTgtReqValueK1 : Inport  Port=6
  Action Port : ActionPort  ActionPortLabel=else
  BrkFuncDataManagement : Stateflow chart  TreatAsAtomicUnit=on
  Bus Selector : BusSelector  OutputSignals=AEBpartFuncImpArb,cycAEBpartFuncImpArb,AEBfullFuncImpArb,JA_AEBfullFuncImpArb,cycAEBfullFuncImpArb,pedAEBfullFuncImpArb,EBAFuncImpArb,FCTBFuncImpArb,JA_PTW_AEBFuncImpArb,JA_cycAEBFuncImpArb,JA_pedAEBFuncImpArb
  Constant : Constant  Value=P_AEB_FIM_VehAEBPartDecelGradientThres
  Constant1 : Constant  Value=P_AEB_FIM_VehAEBPartDecelMinThres
  Constant10 : Constant  Value=P_AEB_FIM_PedAEBfullDecelMinThres
  Constant11 : Constant  Value=P_AEB_FIM_FCTBDecelGradientThres
  Constant12 : Constant  Value=P_AEB_FIM_FCTBDecelMinThres
  Constant13 : Constant  Value=P_AEB_FIM_JAAEBfullDecelGradientThres
  Constant14 : Constant  Value=P_AEB_FIM_JAAEBfullDecelMinThres
  Constant15 : Constant  Value=P_AEB_FIM_VehEBADecelGradientThres
  Constant16 : Constant  Value=P_AEB_FIM_VehEBADecelMinThres
  Constant17 : Constant  Value=P_AEB_FIM_JA_PTW_AEBDecelGradientThres
  Constant18 : Constant  Value=P_AEB_FIM_JA_PTW_AEBDecelMinThres
  Constant19 : Constant  Value=P_AEB_FIM_JA_cycAEBDecelGradientThres
  Constant2 : Constant  Value=P_AEB_FIM_CycAEBPartDecelGradientThres
  Constant20 : Constant  Value=P_AEB_FIM_JA_cycAEBDecelMinThres
  Constant21 : Constant  Value=P_AEB_FIM_JA_pedAEBDecelGradientThres
  Constant22 : Constant  Value=P_AEB_FIM_JA_pedAEBDecelMinThres
  Constant3 : Constant  Value=P_AEB_FIM_BrkControllerConfig
  Constant4 : Constant  Value=P_AEB_FIM_VehAEBfullDecelGradientThresMO
  Constant5 : Constant  Value=P_AEB_FIM_VehAEBfullDecelGradientThresSO
  Constant6 : Constant  Value=P_AEB_FIM_VehAEBfullDecelMinThresSO
  Constant7 : Constant  Value=P_AEB_FIM_CycAEBfullDecelGradientThres
  Constant8 : Constant  Value=P_AEB_FIM_CycAEBfullDecelMinThres
  Constant9 : Constant  Value=P_AEB_FIM_PedAEBfullDecelGradientThres
  Terminator : Terminator
  Terminator1 : Terminator
  axTgtReqValue : Outport
  wires:
  Constant3/1 -> BrkFuncDataManagement/39(P_AEB_FIM_BrkControllerConfig)
  Constant22/1 -> BrkFuncDataManagement/38(P_FIM_JA_pedAEBDecelMinThres)
  Constant21/1 -> BrkFuncDataManagement/37(P_FIM_JA_pedAEBDecelGradientThres)
  Constant20/1 -> BrkFuncDataManagement/36(P_FIM_JA_cycAEBDecelMinThres)
  Constant19/1 -> BrkFuncDataManagement/35(P_FIM_JA_cycAEBDecelGradientThres)
  Constant18/1 -> BrkFuncDataManagement/34(P_FIM_JA_PTW_AEBDecelMinThres)
  Constant17/1 -> BrkFuncDataManagement/33(P_FIM_JA_PTW_AEBDecelGradientThres)
  Constant16/1 -> BrkFuncDataManagement/32(P_FIM_VehEBADecelMinThres)
  Constant15/1 -> BrkFuncDataManagement/31(P_FIM_VehEBADecelGradientThres)
  Constant14/1 -> BrkFuncDataManagement/30(P_FIM_JAAEBfullDecelMinThres)
  Constant13/1 -> BrkFuncDataManagement/29(P_FIM_JAAEBfullDecelGradientThres)
  Constant12/1 -> BrkFuncDataManagement/28(P_FIM_FCTBDecelMinThres)
  Constant11/1 -> BrkFuncDataManagement/27(P_FIM_FCTBDecelGradientThres)
  Constant10/1 -> BrkFuncDataManagement/26(P_FIM_PedAEBfullDecelMinThres)
  Constant9/1 -> BrkFuncDataManagement/25(P_FIM_PedAEBfullDecelGradientThres)
  Constant8/1 -> BrkFuncDataManagement/24(P_FIM_CycAEBfullDecelMinThres)
  Constant7/1 -> BrkFuncDataManagement/23(P_FIM_CycAEBfullDecelGradientThres)
  Constant6/1 -> BrkFuncDataManagement/22(P_FIM_VehAEBfullDecelMinThresSO)
  Constant5/1 -> BrkFuncDataManagement/21(P_FIM_VehAEBfullDecelGradientThresSO)
  Constant4/1 -> BrkFuncDataManagement/20(P_FIM_VehAEBfullDecelGradientThresMO)
  Constant2/1 -> BrkFuncDataManagement/19(P_FIM_CycAEBPartDecelGradientThres)
  Constant1/1 -> BrkFuncDataManagement/18(P_FIM_VehAEBPartDecelMinThres)
  Constant/1 -> BrkFuncDataManagement/17(P_FIM_VehAEBPartDecelGradientThres)
  ObjectMovingState/1 -> BrkFuncDataManagement/13(longTgt_MovingState)
  axTgtReqValueK1/1 -> BrkFuncDataManagement/16(axTgtReqValueK1)  "axTgtReqValueK1"
  FCTB_BrkgReqVal/1 -> BrkFuncDataManagement/15(FCTB_BrkgReqVal)
  AEB_FctbIsNeedBrake/1 -> BrkFuncDataManagement/14(IsNeedBrake)
  subjVel/1 -> BrkFuncDataManagement/12(subjVel)
  Bus Selector/8 -> BrkFuncDataManagement/8(FCTBFuncImpArb)  "<FCTBFuncImpArb>"
  Bus Selector/6 -> BrkFuncDataManagement/6(pedAEBfullFuncImpArb)  "<pedAEBfullFuncImpArb>"
  AEBModStsFuncImpArb/1 -> Bus Selector/1
  Bus Selector/11 -> BrkFuncDataManagement/11(JA_pedAEBFuncImpArb)  "<JA_pedAEBFuncImpArb>"
  Bus Selector/9 -> BrkFuncDataManagement/9(JA_PTW_AEBFuncImpArb)  "<JA_PTW_AEBFuncImpArb>"
  Bus Selector/5 -> BrkFuncDataManagement/5(cycAEBfullFuncImpArb)  "<cycAEBfullFuncImpArb>"
  Bus Selector/7 -> BrkFuncDataManagement/7(EBAFuncImpArb)  "<EBAFuncImpArb>"
  Bus Selector/2 -> BrkFuncDataManagement/2(cycAEBpartFuncImpArb)  "<cycAEBpartFuncImpArb>"
  Bus Selector/10 -> BrkFuncDataManagement/10(JA_cycAEBFuncImpArb)  "<JA_cycAEBFuncImpArb>"
  Bus Selector/3 -> BrkFuncDataManagement/3(AEBfullFuncImpArb)  "<AEBfullFuncImpArb>"
  Bus Selector/4 -> BrkFuncDataManagement/4(JA_AEBfullFuncImpArb)  "<JA_AEBfullFuncImpArb>"
  Bus Selector/1 -> BrkFuncDataManagement/1(AEBpartFuncImpArb)  "<AEBpartFuncImpArb>"
  BrkFuncDataManagement/1(maxDecelGredient) -> Terminator1/1
  BrkFuncDataManagement/2(maxDecel) -> Terminator/1
  BrkFuncDataManagement/3(axTgtReqValue) -> axTgtReqValue/1

== system TosAeb/FcwAebJa_Step_sys/ALG_AEBSystem/ALG_AEBController/AEB_FuncImpManagementApp/FuncManeuArbCalc/brkFuncManeuArbCalc/axTgtReqValueManeuCalc/AEBQuitFlgJudge ==
  AEBTrigger : Inport
  Compare To Constant : simulink/Logic and Bit Operations/Compare To Constant  relop===  const=FALSE  OutDataTypeStr=boolean
  Compare To Constant1 : simulink/Logic and Bit Operations/Compare To Constant  relop===  const=TRUE  OutDataTypeStr=boolean
  Logical Operator : Logic  AllPortsSameDT=off  OutDataTypeStr=boolean
  Unit Delay : UnitDelay  SampleTime=-1  HasFrameUpgradeWarning=on
  AEBQuitFlg : Outport
  wires:
  Unit Delay/1 -> Compare To Constant1/1
  Compare To Constant1/1 -> Logical Operator/2
  AEBTrigger/1 -> Unit Delay/1, Compare To Constant/1
  Compare To Constant/1 -> Logical Operator/1
  Logical Operator/1 -> AEBQuitFlg/1  "AEBQuitFlg"

== system TosAeb/FcwAebJa_Step_sys/ALG_AEBSystem/ALG_AEBController/AEB_FuncImpManagementApp/FuncManeuArbCalc/brkFuncManeuArbCalc/axTgtReqValueManeuCalc/AEBTriggerManeuCalc/axTgtReqValueOptCalc ==
  Ts : Inport  OutDataTypeStr=single
  axJerkQuit : Inport  Port=2  OutDataTypeStr=single
  axTgtReqValueManeuK1 : Inport  Port=3  OutDataTypeStr=single
  f : TriggerPort  TriggerType=function-call  VariantControl=Variant
      settings: CopyFcn=Stateflow.SLINSF.SimulinkMan.removeBlockPreDeleteFcn  PreDeleteFcn=Stateflow.SLINSF.SimulinkMan.unsafePredeleteFcn
  Add : Sum  OutDataTypeStr=single
  Constant4 : Constant  Value=CNNull  OutDataTypeStr=single
  MinMax : MinMax  Inputs=2  OutDataTypeStr=single
  Product : Product  OutDataTypeStr=single
  axTgtReqValueManeu : Outport
  wires:
  axTgtReqValueManeuK1/1 -> Add/2
  MinMax/1 -> axTgtReqValueManeu/1
  Add/1 -> MinMax/1
  Ts/1 -> Product/1
  axJerkQuit/1 -> Product/2
  Constant4/1 -> MinMax/2
  Product/1 -> Add/1

== system TosAeb/FcwAebJa_Step_sys/ALG_AEBSystem/ALG_AEBController/AEB_FuncImpManagementApp/FuncManeuArbCalc/brkFuncManeuArbCalc/axTgtReqValueManeuCalc/AEBTriggerManeuCalc1/axTgtReqValueOptCalc ==
  Ts : Inport  OutDataTypeStr=single
  axJerkQuit : Inport  Port=2  OutDataTypeStr=single
  axTgtReqValueIntial : Inport  Port=3  OutDataTypeStr=single
  f : TriggerPort  TriggerType=function-call  VariantControl=Variant
      settings: CopyFcn=Stateflow.SLINSF.SimulinkMan.removeBlockPreDeleteFcn  PreDeleteFcn=Stateflow.SLINSF.SimulinkMan.unsafePredeleteFcn
  Add : Sum  OutDataTypeStr=single
  Constant4 : Constant  Value=CNNull  OutDataTypeStr=single
  Delay : Delay  DelayLength=1  InitialConditionSource=Input port
      settings: InputPortMap=u0,p4
  MinMax : MinMax  Inputs=2  OutDataTypeStr=single
  Product : Product  OutDataTypeStr=single
  axTgtReqValueManeu : Outport
  wires:
  Product/1 -> Add/1
  Constant4/1 -> MinMax/2
  Delay/1 -> Add/2
  axJerkQuit/1 -> Product/2
  Ts/1 -> Product/1
  Add/1 -> MinMax/1
  MinMax/1 -> axTgtReqValueManeu/1, Delay/1
  axTgtReqValueIntial/1 -> Delay/2

== system TosAeb/FcwAebJa_Step_sys/ALG_AEBSystem/ALG_AEBController/AEB_FuncImpManagementApp/FuncManeuArbCalc/brkFuncManeuArbCalc/brkManeuAborCalc/DgrCondCalc ==
  ObjectMovingState : Inport
  axAbsObj : Inport  Port=2
  accelAvoid : Inport  Port=3
  Compare To Constant : simulink/Logic and Bit Operations/Compare To Constant  relop=>  const=P_AEB_FIM_DangerCalObjAccelThres  OutDataTypeStr=boolean
  Compare To Constant1 : simulink/Logic and Bit Operations/Compare To Constant  relop===  const=AEB_ObjectMovingState.Moving  OutDataTypeStr=boolean
  Compare To Constant2 : simulink/Logic and Bit Operations/Compare To Constant  relop=>  const=P_AEB_FIM_DangerCalAccelAvoidThres  OutDataTypeStr=boolean
  Constant : Constant  Value=FALSE
  Logical Operator : Logic  AllPortsSameDT=off  OutDataTypeStr=boolean
  Switch : Switch  Criteria=u2 ~= 0
  dgrCond : Outport
  wires:
  Logical Operator/1 -> Switch/2
  Constant/1 -> Switch/3
  Compare To Constant2/1 -> Switch/1
  accelAvoid/1 -> Compare To Constant2/1
  Switch/1 -> dgrCond/1
  axAbsObj/1 -> Compare To Constant/1
  Compare To Constant/1 -> Logical Operator/2
  ObjectMovingState/1 -> Compare To Constant1/1
  Compare To Constant1/1 -> Logical Operator/1

== system TosAeb/FcwAebJa_Step_sys/ALG_AEBSystem/ALG_AEBController/AEB_FuncImpManagementApp/FuncManeuArbCalc/brkFuncManeuArbCalc/brkManeuAborCalc/ESPRelAborCondCalc ==
  AEBBA : Inport
  AEBIB : Inport  Port=2
  ESPRelAborCondFlow : Stateflow chart  TreatAsAtomicUnit=on
  Unit Delay : UnitDelay  SampleTime=-1  HasFrameUpgradeWarning=on
  Unit Delay1 : UnitDelay  SampleTime=-1  HasFrameUpgradeWarning=on
  ESPRelAborCond : Outport
  wires:
  ESPRelAborCondFlow/1(ESPRelAborCond) -> ESPRelAborCond/1
  Unit Delay/1 -> ESPRelAborCondFlow/2(AEBBAK1)
  Unit Delay1/1 -> ESPRelAborCondFlow/4(AEBIBK1)
  AEBIB/1 -> ESPRelAborCondFlow/3(AEBIB), Unit Delay1/1
  AEBBA/1 -> Unit Delay/1, ESPRelAborCondFlow/1(AEBBA)

== system TosAeb/FcwAebJa_Step_sys/ALG_AEBSystem/ALG_AEBController/AEB_FuncImpManagementApp/FuncManeuArbCalc/brkFuncManeuArbCalc/brkManeuAborCalc/JABrkManeuAborCalc ==
  AEBTrigger : Inport
  VelReduceMaxCond : Inport  Port=2
  dgrAborCond : Inport  Port=3
  ESPRelAborCond : Inport  Port=4
  Compare To Constant : simulink/Logic and Bit Operations/Compare To Constant  relop===  const=TRUE  OutDataTypeStr=boolean
  Compare To Constant1 : simulink/Logic and Bit Operations/Compare To Constant  relop===  const=TRUE  OutDataTypeStr=boolean
  Compare To Constant2 : simulink/Logic and Bit Operations/Compare To Constant  relop===  const=TRUE  OutDataTypeStr=boolean
  Logical Operator : Logic  Operator=OR  Inputs=3  AllPortsSameDT=off  OutDataTypeStr=boolean
  Logical Operator1 : Logic  AllPortsSameDT=off  OutDataTypeStr=boolean
  JABrkManeuAbor : Outport
  wires:
  Logical Operator1/1 -> JABrkManeuAbor/1
  AEBTrigger/1 -> Logical Operator1/1
  Logical Operator/1 -> Logical Operator1/2
  Compare To Constant2/1 -> Logical Operator/3
  Compare To Constant1/1 -> Logical Operator/2
  Compare To Constant/1 -> Logical Operator/1
  ESPRelAborCond/1 -> Compare To Constant2/1
  dgrAborCond/1 -> Compare To Constant1/1
  VelReduceMaxCond/1 -> Compare To Constant/1

== system TosAeb/FcwAebJa_Step_sys/ALG_AEBSystem/ALG_AEBController/AEB_FuncImpManagementApp/FuncManeuArbCalc/brkFuncManeuArbCalc/brkManeuAborCalc/LatBrkManeuAborCalc ==
  AEBTrigger : Inport
  VelReduceMaxCond : Inport  Port=2
  dgrAborCond : Inport  Port=3
  ESPRelAborCond : Inport  Port=4
  Compare To Constant : simulink/Logic and Bit Operations/Compare To Constant  relop===  const=TRUE  OutDataTypeStr=boolean
  Compare To Constant1 : simulink/Logic and Bit Operations/Compare To Constant  relop===  const=TRUE  OutDataTypeStr=boolean
  Compare To Constant2 : simulink/Logic and Bit Operations/Compare To Constant  relop===  const=TRUE  OutDataTypeStr=boolean
  Logical Operator : Logic  Operator=OR  Inputs=3  AllPortsSameDT=off  OutDataTypeStr=boolean
  Logical Operator1 : Logic  AllPortsSameDT=off  OutDataTypeStr=boolean
  latBrkManeuAbor : Outport
  wires:
  VelReduceMaxCond/1 -> Compare To Constant/1
  dgrAborCond/1 -> Compare To Constant1/1
  ESPRelAborCond/1 -> Compare To Constant2/1
  Compare To Constant/1 -> Logical Operator/1
  Compare To Constant1/1 -> Logical Operator/2
  Compare To Constant2/1 -> Logical Operator/3
  Logical Operator/1 -> Logical Operator1/2
  AEBTrigger/1 -> Logical Operator1/1
  Logical Operator1/1 -> latBrkManeuAbor/1

== system TosAeb/FcwAebJa_Step_sys/ALG_AEBSystem/ALG_AEBController/AEB_FuncImpManagementApp/FuncManeuArbCalc/brkFuncManeuArbCalc/brkManeuAborCalc/VelCond ==
  note: 添加目标加速度条件，目标处于减速状态，
      | 当相对车速大于0时；功能不退出
  note: P_AEB_FIM_EBAVelDecMaxThres
  vx : Inport
  axAbsObj : Inport  Port=2
  ObjectMovingState : Inport  Port=3
  Compare To Constant : simulink/Logic and Bit Operations/Compare To Constant  relop===  const=AEB_ObjectMovingState.Moving  OutDataTypeStr=boolean
  Compare To Constant1 : simulink/Logic and Bit Operations/Compare To Constant  relop=>  const=P_AEB_FIM_VxAborThres  OutDataTypeStr=boolean
  Compare To Constant2 : simulink/Logic and Bit Operations/Compare To Constant  relop=>  const=P_AEB_FIM_AxAbsObjAborThres  OutDataTypeStr=boolean
  Compare To Constant3 : simulink/Logic and Bit Operations/Compare To Constant  relop=>  const=P_AEB_FIM_VxMOAborThres  OutDataTypeStr=boolean
  Logical Operator : Logic  Inputs=3  AllPortsSameDT=off  OutDataTypeStr=boolean
  Logical Operator1 : Logic  Operator=OR  AllPortsSameDT=off  OutDataTypeStr=boolean
  VelCond : Outport
  wires:
  Compare To Constant3/1 -> Logical Operator/2
  Compare To Constant/1 -> Logical Operator/1
  Compare To Constant1/1 -> Logical Operator1/1
  Logical Operator/1 -> Logical Operator1/2
  Compare To Constant2/1 -> Logical Operator/3
  axAbsObj/1 -> Compare To Constant2/1
  Logical Operator1/1 -> VelCond/1
  vx/1 -> Compare To Constant1/1, Compare To Constant3/1
  ObjectMovingState/1 -> Compare To Constant/1
